simulink model "fft_2p_4kc_18i_18o_core"  (R2021a)
settings: Solver=VariableStepDiscrete  StartTime=0.0  StopTime=2^11*3  RelTol=1e-3
undefined symbols (not defined in the file or in its folder): i
libraries: xbsIndex_r4 x48628, casper_library_misc x100, casper_library_delays x10
(part 153 of 338)

== system fft_2p_4kc_18i_18o_core/fft/fft_biplex/biplex_core/fft_stage_3/butterfly_direct/bus_add ==
  a : Inport
  b : Inport  Port=2
  a_debus : Subsystem  AncestorBlock=casper_library_flow_control/bus_expand  mask(bus_expand){mode=divisions of arbitrary size, outputNum=2, outputWidth=[18  18  18  18  18  18  18  18  18  18  18  18  18  18  18  18  18  18  18  18  18  18  18  18  18  18  18  18  18  18  18  18], outputBinaryPt=[17  17  17  17  17  17  17  17  17  17  17  17  17  17  17  17  17  17  17  17  17  17  17  17  17  17  17  17  17  17  17  17], outputArithmeticType=[1  1  1  1  1  1  1  1  1  1  1  1  1  1  1  1  1  1  1  1  1  1  1  1  1  1  1  1  1  1  1  1], show_format=on, outputToWorkspace=off, variablePrefix=, outputToModelAsWell=on}
      mask prompts: outputNum "Number of outputs:"; outputWidth "Output width:"; outputBinaryPt "Output binary point position:"; outputArithmeticType "Output arithmetic type (ufix=0, fix=1, bool=2):"; show_format "Print format string?"; outputToWorkspace "Output to workspace?"; variablePrefix "Variable name prefix:"; outputToModelAsWell "Output to model as well?"
      mask help:
      | Expands a 'bus' made using a bus_create or Xilinx
      | concat block.
      | The input will be divided into the specified number
      | of outputs with the specified arithmetic types.
      | Divisions are made from most significant bit.
      mask init: same as fft_2p_4kc_18i_18o_core/bus_expand
      settings: AttributesFormatString=32 outputs: f18.17,f18.17,f18.17,f18.17,f18.17,f18.17,f18.17,f18.17,f18.17,f18.17,f18.17,f18.17,f18.17,f18.17,f18.17,f18.17,f18.17,f18.17,f18.17,f18.17,f18.17,f18.17,f18.17,f18.17,f18.17,f18.17,f18.17,f18.17,f18.17,f18.17,f18.17,f18.17,
      mask TypeOptions mode: divisions of equal size | divisions of arbitrary size
      mask Callback mode: %bus_expand_callback();
      mask Callback outputWidth: %bus_expand_callback();
      mask Callback outputToWorkspace: %bus_expand_callback();
  addsub{1..32} (32 alike) : xbsIndex_r4/AddSub  mode=Addition  use_carryin=off  use_carryout=off  en=off  latency=1  precision=User Defined  arith_type="Signed  (2's comp)"  n_bits=20  bin_pt=17  quantization=Truncate  overflow=Wrap  use_behavioral_HDL=on  hw_selection=Fabric  pipelined=on  dbl_ovrd=off  block_type=addsub
  b_debus : Subsystem  AncestorBlock=casper_library_flow_control/bus_expand  mask(bus_expand){mode=divisions of arbitrary size, outputNum=2, outputWidth=[19  19  19  19  19  19  19  19  19  19  19  19  19  19  19  19  19  19  19  19  19  19  19  19  19  19  19  19  19  19  19  19], outputBinaryPt=[17  17  17  17  17  17  17  17  17  17  17  17  17  17  17  17  17  17  17  17  17  17  17  17  17  17  17  17  17  17  17  17], outputArithmeticType=[1  1  1  1  1  1  1  1  1  1  1  1  1  1  1  1  1  1  1  1  1  1  1  1  1  1  1  1  1  1  1  1], show_format=on, outputToWorkspace=off, variablePrefix=, outputToModelAsWell=on}
      mask prompts: outputNum "Number of outputs:"; outputWidth "Output width:"; outputBinaryPt "Output binary point position:"; outputArithmeticType "Output arithmetic type (ufix=0, fix=1, bool=2):"; show_format "Print format string?"; outputToWorkspace "Output to workspace?"; variablePrefix "Variable name prefix:"; outputToModelAsWell "Output to model as well?"
      mask help:
      | Expands a 'bus' made using a bus_create or Xilinx
      | concat block.
      | The input will be divided into the specified number
      | of outputs with the specified arithmetic types.
      | Divisions are made from most significant bit.
      mask init: same as fft_2p_4kc_18i_18o_core/bus_expand
      settings: AttributesFormatString=32 outputs: f19.17,f19.17,f19.17,f19.17,f19.17,f19.17,f19.17,f19.17,f19.17,f19.17,f19.17,f19.17,f19.17,f19.17,f19.17,f19.17,f19.17,f19.17,f19.17,f19.17,f19.17,f19.17,f19.17,f19.17,f19.17,f19.17,f19.17,f19.17,f19.17,f19.17,f19.17,f19.17,
      mask TypeOptions mode: divisions of equal size | divisions of arbitrary size
      mask Callback mode: %bus_expand_callback();
      mask Callback outputWidth: %bus_expand_callback();
      mask Callback outputToWorkspace: %bus_expand_callback();
  op_bussify : Subsystem  AncestorBlock=casper_library_flow_control/bus_create  mask(bus_create){inputNum=32}
      mask prompts: inputNum "Number of inputs:"
      mask init:
      | bus_create_init(gcb,...
      |     'inputNum', inputNum);
  dout : Outport
  wires:
  a/1 -> a_debus/1(bus_in)
  b/1 -> b_debus/1(bus_in)
  a_debus/1(msb_out32) -> addsub1/1
  b_debus/1(msb_out32) -> addsub1/2
  a_debus/2(out31) -> addsub2/1
  b_debus/2(out31) -> addsub2/2
  a_debus/3(out30) -> addsub3/1
  b_debus/3(out30) -> addsub3/2
  a_debus/4(out29) -> addsub4/1
  b_debus/4(out29) -> addsub4/2
  a_debus/5(out28) -> addsub5/1
  b_debus/5(out28) -> addsub5/2
  a_debus/6(out27) -> addsub6/1
  b_debus/6(out27) -> addsub6/2
  a_debus/7(out26) -> addsub7/1
  b_debus/7(out26) -> addsub7/2
  a_debus/8(out25) -> addsub8/1
  b_debus/8(out25) -> addsub8/2
  a_debus/9(out24) -> addsub9/1
  b_debus/9(out24) -> addsub9/2
  a_debus/10(out23) -> addsub10/1
  b_debus/10(out23) -> addsub10/2
  a_debus/11(out22) -> addsub11/1
  b_debus/11(out22) -> addsub11/2
  a_debus/12(out21) -> addsub12/1
  b_debus/12(out21) -> addsub12/2
  a_debus/13(out20) -> addsub13/1
  b_debus/13(out20) -> addsub13/2
  a_debus/14(out19) -> addsub14/1
  b_debus/14(out19) -> addsub14/2
  a_debus/15(out18) -> addsub15/1
  b_debus/15(out18) -> addsub15/2
  a_debus/16(out17) -> addsub16/1
  b_debus/16(out17) -> addsub16/2
  a_debus/17(out16) -> addsub17/1
  b_debus/17(out16) -> addsub17/2
  a_debus/18(out15) -> addsub18/1
  b_debus/18(out15) -> addsub18/2
  a_debus/19(out14) -> addsub19/1
  b_debus/19(out14) -> addsub19/2
  a_debus/20(out13) -> addsub20/1
  b_debus/20(out13) -> addsub20/2
  a_debus/21(out12) -> addsub21/1
  b_debus/21(out12) -> addsub21/2
  a_debus/22(out11) -> addsub22/1
  b_debus/22(out11) -> addsub22/2
  a_debus/23(out10) -> addsub23/1
  b_debus/23(out10) -> addsub23/2
  a_debus/24(out9) -> addsub24/1
  b_debus/24(out9) -> addsub24/2
  a_debus/25(out8) -> addsub25/1
  b_debus/25(out8) -> addsub25/2
  a_debus/26(out7) -> addsub26/1
  b_debus/26(out7) -> addsub26/2
  a_debus/27(out6) -> addsub27/1
  b_debus/27(out6) -> addsub27/2
  a_debus/28(out5) -> addsub28/1
  b_debus/28(out5) -> addsub28/2
  a_debus/29(out4) -> addsub29/1
  b_debus/29(out4) -> addsub29/2
  a_debus/30(out3) -> addsub30/1
  b_debus/30(out3) -> addsub30/2
  a_debus/31(out2) -> addsub31/1
  b_debus/31(out2) -> addsub31/2
  a_debus/32(lsb_out1) -> addsub32/1
  b_debus/32(lsb_out1) -> addsub32/2
  addsub1/1 -> op_bussify/1(in1)
  addsub2/1 -> op_bussify/2(in2)
  addsub3/1 -> op_bussify/3(in3)
  addsub4/1 -> op_bussify/4(in4)
  addsub5/1 -> op_bussify/5(in5)
  addsub6/1 -> op_bussify/6(in6)
  addsub7/1 -> op_bussify/7(in7)
  addsub8/1 -> op_bussify/8(in8)
  addsub9/1 -> op_bussify/9(in9)
  addsub10/1 -> op_bussify/10(in10)
  addsub11/1 -> op_bussify/11(in11)
  addsub12/1 -> op_bussify/12(in12)
  addsub13/1 -> op_bussify/13(in13)
  addsub14/1 -> op_bussify/14(in14)
  addsub15/1 -> op_bussify/15(in15)
  addsub16/1 -> op_bussify/16(in16)
  addsub17/1 -> op_bussify/17(in17)
  addsub18/1 -> op_bussify/18(in18)
  addsub19/1 -> op_bussify/19(in19)
  addsub20/1 -> op_bussify/20(in20)
  addsub21/1 -> op_bussify/21(in21)
  addsub22/1 -> op_bussify/22(in22)
  addsub23/1 -> op_bussify/23(in23)
  addsub24/1 -> op_bussify/24(in24)
  addsub25/1 -> op_bussify/25(in25)
  addsub26/1 -> op_bussify/26(in26)
  addsub27/1 -> op_bussify/27(in27)
  addsub28/1 -> op_bussify/28(in28)
  addsub29/1 -> op_bussify/29(in29)
  addsub30/1 -> op_bussify/30(in30)
  addsub31/1 -> op_bussify/31(in31)
  addsub32/1 -> op_bussify/32(in32)
  op_bussify/1(bus_out) -> dout/1
